simulink model "r2_c8n856m1k_vacc"  (R2013a)
settings: Solver=FixedStepDiscrete  SolverMode=SingleTasking  StartTime=0.0  StopTime=10000  RelTol=1e-3
undefined symbols (not defined in the file): Down, Up
libraries: xbsIndex_r4 x32309, casper_library_misc x83, casper_library_delays x13, casper_library_communications x8, xbsReplacements_r4 x3, xps_library x2
(part 49 of 251)

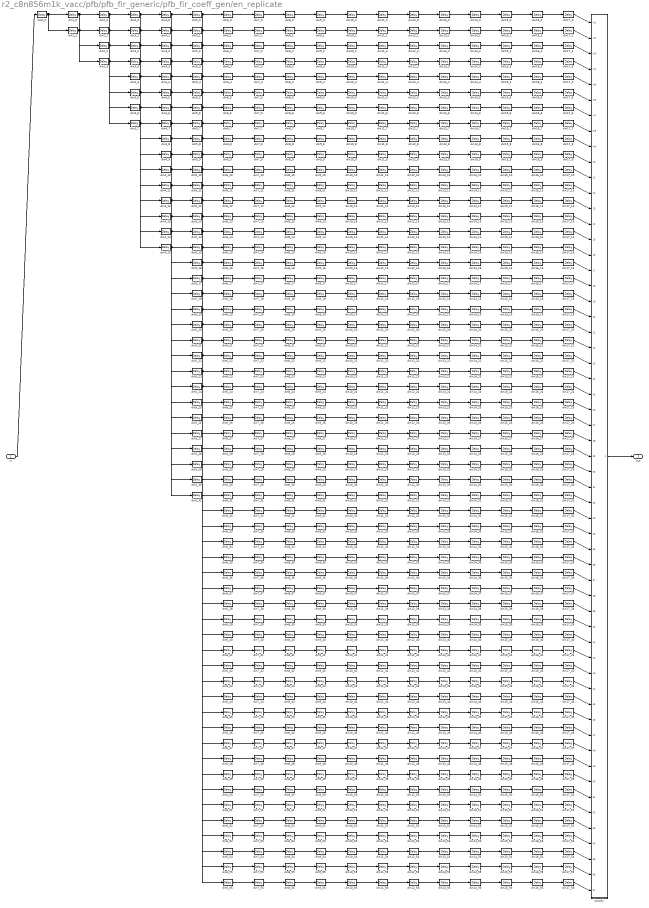
== system r2_c8n856m1k_vacc/pfb/pfb_fir_generic/pfb_fir_coeff_gen/en_replicate ==
  in : Inport
  bussify : Subsystem  AncestorBlock=casper_library_flow_control/bus_create  mask(bus_create){inputNum=57}
      mask prompts: inputNum "Number of inputs:"
      mask init:
      | bus_create_init(gcb,...
      |     'inputNum', inputNum);
  din0_0 : xbsIndex_r4/Delay  rst=off  infoeditControl=Selection of Reset will increase slice count due to use of real FFs and instead of SRLs  en=off  latency=1  dbl_ovrd=off  reg_retiming=off  block_type=delay
  din10_{0..56} (57 alike) : xbsIndex_r4/Delay  rst=off  infoeditControl=Selection of Reset will increase slice count due to use of real FFs and instead of SRLs  en=off  latency=1  dbl_ovrd=off  reg_retiming=on  block_type=delay
  din11_{0..56} (57 alike) : xbsIndex_r4/Delay  rst=off  infoeditControl=Selection of Reset will increase slice count due to use of real FFs and instead of SRLs  en=off  latency=1  dbl_ovrd=off  reg_retiming=on  block_type=delay
  din12_{0..56} (57 alike) : xbsIndex_r4/Delay  rst=off  infoeditControl=Selection of Reset will increase slice count due to use of real FFs and instead of SRLs  en=off  latency=1  dbl_ovrd=off  reg_retiming=on  block_type=delay
  din13_{0..56} (57 alike) : xbsIndex_r4/Delay  rst=off  infoeditControl=Selection of Reset will increase slice count due to use of real FFs and instead of SRLs  en=off  latency=1  dbl_ovrd=off  reg_retiming=on  block_type=delay
  din14_{0..56} (57 alike) : xbsIndex_r4/Delay  rst=off  infoeditControl=Selection of Reset will increase slice count due to use of real FFs and instead of SRLs  en=off  latency=1  dbl_ovrd=off  reg_retiming=on  block_type=delay
  din15_{0..56} (57 alike) : xbsIndex_r4/Delay  rst=off  infoeditControl=Selection of Reset will increase slice count due to use of real FFs and instead of SRLs  en=off  latency=1  dbl_ovrd=off  reg_retiming=on  block_type=delay
  din16_{0..56} (57 alike) : xbsIndex_r4/Delay  rst=off  infoeditControl=Selection of Reset will increase slice count due to use of real FFs and instead of SRLs  en=off  latency=1  dbl_ovrd=off  reg_retiming=on  block_type=delay
  din17_{0..56} (57 alike) : xbsIndex_r4/Delay  rst=off  infoeditControl=Selection of Reset will increase slice count due to use of real FFs and instead of SRLs  en=off  latency=1  dbl_ovrd=off  reg_retiming=off  block_type=delay
  din1_0 : xbsIndex_r4/Delay  rst=off  infoeditControl=Selection of Reset will increase slice count due to use of real FFs and instead of SRLs  en=off  latency=1  dbl_ovrd=off  reg_retiming=off  block_type=delay
  din1_1 : xbsIndex_r4/Delay  rst=off  infoeditControl=Selection of Reset will increase slice count due to use of real FFs and instead of SRLs  en=off  latency=1  dbl_ovrd=off  reg_retiming=off  block_type=delay
  din2_{0..3} (4 alike) : xbsIndex_r4/Delay  rst=off  infoeditControl=Selection of Reset will increase slice count due to use of real FFs and instead of SRLs  en=off  latency=1  dbl_ovrd=off  reg_retiming=off  block_type=delay
  din3_{0..7} (8 alike) : xbsIndex_r4/Delay  rst=off  infoeditControl=Selection of Reset will increase slice count due to use of real FFs and instead of SRLs  en=off  latency=1  dbl_ovrd=off  reg_retiming=off  block_type=delay
  din4_{0..15} (16 alike) : xbsIndex_r4/Delay  rst=off  infoeditControl=Selection of Reset will increase slice count due to use of real FFs and instead of SRLs  en=off  latency=1  dbl_ovrd=off  reg_retiming=off  block_type=delay
  din5_{0..31} (32 alike) : xbsIndex_r4/Delay  rst=off  infoeditControl=Selection of Reset will increase slice count due to use of real FFs and instead of SRLs  en=off  latency=1  dbl_ovrd=off  reg_retiming=off  block_type=delay
  din6_{0..56} (57 alike) : xbsIndex_r4/Delay  rst=off  infoeditControl=Selection of Reset will increase slice count due to use of real FFs and instead of SRLs  en=off  latency=1  dbl_ovrd=off  reg_retiming=on  block_type=delay
  din7_{0..56} (57 alike) : xbsIndex_r4/Delay  rst=off  infoeditControl=Selection of Reset will increase slice count due to use of real FFs and instead of SRLs  en=off  latency=1  dbl_ovrd=off  reg_retiming=on  block_type=delay
  din8_{0..56} (57 alike) : xbsIndex_r4/Delay  rst=off  infoeditControl=Selection of Reset will increase slice count due to use of real FFs and instead of SRLs  en=off  latency=1  dbl_ovrd=off  reg_retiming=on  block_type=delay
  din9_{0..56} (57 alike) : xbsIndex_r4/Delay  rst=off  infoeditControl=Selection of Reset will increase slice count due to use of real FFs and instead of SRLs  en=off  latency=1  dbl_ovrd=off  reg_retiming=on  block_type=delay
  out : Outport
  wires:
  in/1 -> din0_0/1
  din0_0/1 -> din1_0/1, din1_1/1
  din1_0/1 -> din2_0/1, din2_2/1
  din1_1/1 -> din2_1/1, din2_3/1
  din2_0/1 -> din3_0/1, din3_4/1
  din2_1/1 -> din3_1/1, din3_5/1
  din2_2/1 -> din3_2/1, din3_6/1
  din2_3/1 -> din3_3/1, din3_7/1
  din3_0/1 -> din4_0/1, din4_8/1
  din3_1/1 -> din4_1/1, din4_9/1
  din3_2/1 -> din4_2/1, din4_10/1
  din3_3/1 -> din4_3/1, din4_11/1
  din3_4/1 -> din4_4/1, din4_12/1
  din3_5/1 -> din4_5/1, din4_13/1
  din3_6/1 -> din4_6/1, din4_14/1
  din3_7/1 -> din4_7/1, din4_15/1
  din4_0/1 -> din5_0/1, din5_16/1
  din4_1/1 -> din5_1/1, din5_17/1
  din4_2/1 -> din5_2/1, din5_18/1
  din4_3/1 -> din5_3/1, din5_19/1
  din4_4/1 -> din5_4/1, din5_20/1
  din4_5/1 -> din5_5/1, din5_21/1
  din4_6/1 -> din5_6/1, din5_22/1
  din4_7/1 -> din5_7/1, din5_23/1
  din4_8/1 -> din5_8/1, din5_24/1
  din4_9/1 -> din5_9/1, din5_25/1
  din4_10/1 -> din5_10/1, din5_26/1
  din4_11/1 -> din5_11/1, din5_27/1
  din4_12/1 -> din5_12/1, din5_28/1
  din4_13/1 -> din5_13/1, din5_29/1
  din4_14/1 -> din5_14/1, din5_30/1
  din4_15/1 -> din5_15/1, din5_31/1
  din5_0/1 -> din6_0/1, din6_32/1
  din5_1/1 -> din6_1/1, din6_33/1
  din5_2/1 -> din6_2/1, din6_34/1
  din5_3/1 -> din6_3/1, din6_35/1
  din5_4/1 -> din6_4/1, din6_36/1
  din5_5/1 -> din6_5/1, din6_37/1
  din5_6/1 -> din6_6/1, din6_38/1
  din5_7/1 -> din6_7/1, din6_39/1
  din5_8/1 -> din6_8/1, din6_40/1
  din5_9/1 -> din6_9/1, din6_41/1
  din5_10/1 -> din6_10/1, din6_42/1
  din5_11/1 -> din6_11/1, din6_43/1
  din5_12/1 -> din6_12/1, din6_44/1
  din5_13/1 -> din6_13/1, din6_45/1
  din5_14/1 -> din6_14/1, din6_46/1
  din5_15/1 -> din6_15/1, din6_47/1
  din5_16/1 -> din6_16/1, din6_48/1
  din5_17/1 -> din6_17/1, din6_49/1
  din5_18/1 -> din6_18/1, din6_50/1
  din5_19/1 -> din6_19/1, din6_51/1
  din5_20/1 -> din6_20/1, din6_52/1
  din5_21/1 -> din6_21/1, din6_53/1
  din5_22/1 -> din6_22/1, din6_54/1
  din5_23/1 -> din6_23/1, din6_55/1
  din5_24/1 -> din6_24/1, din6_56/1
  din5_25/1 -> din6_25/1
  din5_26/1 -> din6_26/1
  din5_27/1 -> din6_27/1
  din5_28/1 -> din6_28/1
  din5_29/1 -> din6_29/1
  din5_30/1 -> din6_30/1
  din5_31/1 -> din6_31/1
  din6_0/1 -> din7_0/1
  din6_1/1 -> din7_1/1
  din6_2/1 -> din7_2/1
  din6_3/1 -> din7_3/1
  din6_4/1 -> din7_4/1
  din6_5/1 -> din7_5/1
  din6_6/1 -> din7_6/1
  din6_7/1 -> din7_7/1
  din6_8/1 -> din7_8/1
  din6_9/1 -> din7_9/1
  din6_10/1 -> din7_10/1
  din6_11/1 -> din7_11/1
  din6_12/1 -> din7_12/1
  din6_13/1 -> din7_13/1
  din6_14/1 -> din7_14/1
  din6_15/1 -> din7_15/1
  din6_16/1 -> din7_16/1
  din6_17/1 -> din7_17/1
  din6_18/1 -> din7_18/1
  din6_19/1 -> din7_19/1
  din6_20/1 -> din7_20/1
  din6_21/1 -> din7_21/1
  din6_22/1 -> din7_22/1
  din6_23/1 -> din7_23/1
  din6_24/1 -> din7_24/1
  din6_25/1 -> din7_25/1
  din6_26/1 -> din7_26/1
  din6_27/1 -> din7_27/1
  din6_28/1 -> din7_28/1
  din6_29/1 -> din7_29/1
  din6_30/1 -> din7_30/1
  din6_31/1 -> din7_31/1
  din6_32/1 -> din7_32/1
  din6_33/1 -> din7_33/1
  din6_34/1 -> din7_34/1
  din6_35/1 -> din7_35/1
  din6_36/1 -> din7_36/1
  din6_37/1 -> din7_37/1
  din6_38/1 -> din7_38/1
  din6_39/1 -> din7_39/1
  din6_40/1 -> din7_40/1
  din6_41/1 -> din7_41/1
  din6_42/1 -> din7_42/1
  din6_43/1 -> din7_43/1
  din6_44/1 -> din7_44/1
  din6_45/1 -> din7_45/1
  din6_46/1 -> din7_46/1
  din6_47/1 -> din7_47/1
  din6_48/1 -> din7_48/1
  din6_49/1 -> din7_49/1
  din6_50/1 -> din7_50/1
  din6_51/1 -> din7_51/1
  din6_52/1 -> din7_52/1
  din6_53/1 -> din7_53/1
  din6_54/1 -> din7_54/1
  din6_55/1 -> din7_55/1
  din6_56/1 -> din7_56/1
  din7_0/1 -> din8_0/1
  din7_1/1 -> din8_1/1
  din7_2/1 -> din8_2/1
  din7_3/1 -> din8_3/1
  din7_4/1 -> din8_4/1
  din7_5/1 -> din8_5/1
  din7_6/1 -> din8_6/1
  din7_7/1 -> din8_7/1
  din7_8/1 -> din8_8/1
  din7_9/1 -> din8_9/1
  din7_10/1 -> din8_10/1
  din7_11/1 -> din8_11/1
  din7_12/1 -> din8_12/1
  din7_13/1 -> din8_13/1
  din7_14/1 -> din8_14/1
  din7_15/1 -> din8_15/1
  din7_16/1 -> din8_16/1
  din7_17/1 -> din8_17/1
  din7_18/1 -> din8_18/1
  din7_19/1 -> din8_19/1
  din7_20/1 -> din8_20/1
  din7_21/1 -> din8_21/1
  din7_22/1 -> din8_22/1
  din7_23/1 -> din8_23/1
  din7_24/1 -> din8_24/1
  din7_25/1 -> din8_25/1
  din7_26/1 -> din8_26/1
  din7_27/1 -> din8_27/1
  din7_28/1 -> din8_28/1
  din7_29/1 -> din8_29/1
  din7_30/1 -> din8_30/1
  din7_31/1 -> din8_31/1
  din7_32/1 -> din8_32/1
  din7_33/1 -> din8_33/1
  din7_34/1 -> din8_34/1
  din7_35/1 -> din8_35/1
  din7_36/1 -> din8_36/1
  din7_37/1 -> din8_37/1
  din7_38/1 -> din8_38/1
  din7_39/1 -> din8_39/1
  din7_40/1 -> din8_40/1
  din7_41/1 -> din8_41/1
  din7_42/1 -> din8_42/1
  din7_43/1 -> din8_43/1
  din7_44/1 -> din8_44/1
  din7_45/1 -> din8_45/1
  din7_46/1 -> din8_46/1
  din7_47/1 -> din8_47/1
  din7_48/1 -> din8_48/1
  din7_49/1 -> din8_49/1
  din7_50/1 -> din8_50/1
  din7_51/1 -> din8_51/1
  din7_52/1 -> din8_52/1
  din7_53/1 -> din8_53/1
  din7_54/1 -> din8_54/1
  din7_55/1 -> din8_55/1
  din7_56/1 -> din8_56/1
  din8_0/1 -> din9_0/1
  din8_1/1 -> din9_1/1
  din8_2/1 -> din9_2/1
  din8_3/1 -> din9_3/1
  din8_4/1 -> din9_4/1
  din8_5/1 -> din9_5/1
  din8_6/1 -> din9_6/1
  din8_7/1 -> din9_7/1
  din8_8/1 -> din9_8/1
  din8_9/1 -> din9_9/1
  din8_10/1 -> din9_10/1
  din8_11/1 -> din9_11/1
  din8_12/1 -> din9_12/1
  din8_13/1 -> din9_13/1
  din8_14/1 -> din9_14/1
  din8_15/1 -> din9_15/1
  din8_16/1 -> din9_16/1
  din8_17/1 -> din9_17/1
  din8_18/1 -> din9_18/1
  din8_19/1 -> din9_19/1
  din8_20/1 -> din9_20/1
  din8_21/1 -> din9_21/1
  din8_22/1 -> din9_22/1
  din8_23/1 -> din9_23/1
  din8_24/1 -> din9_24/1
  din8_25/1 -> din9_25/1
  din8_26/1 -> din9_26/1
  din8_27/1 -> din9_27/1
  din8_28/1 -> din9_28/1
  din8_29/1 -> din9_29/1
  din8_30/1 -> din9_30/1
  din8_31/1 -> din9_31/1
  din8_32/1 -> din9_32/1
  din8_33/1 -> din9_33/1
  din8_34/1 -> din9_34/1
  din8_35/1 -> din9_35/1
  din8_36/1 -> din9_36/1
  din8_37/1 -> din9_37/1
  din8_38/1 -> din9_38/1
  din8_39/1 -> din9_39/1
  din8_40/1 -> din9_40/1
  din8_41/1 -> din9_41/1
  din8_42/1 -> din9_42/1
  din8_43/1 -> din9_43/1
  din8_44/1 -> din9_44/1
  din8_45/1 -> din9_45/1
  din8_46/1 -> din9_46/1
  din8_47/1 -> din9_47/1
  din8_48/1 -> din9_48/1
  din8_49/1 -> din9_49/1
  din8_50/1 -> din9_50/1
  din8_51/1 -> din9_51/1
  din8_52/1 -> din9_52/1
  din8_53/1 -> din9_53/1
  din8_54/1 -> din9_54/1
  din8_55/1 -> din9_55/1
  din8_56/1 -> din9_56/1
  din9_0/1 -> din10_0/1
  din9_1/1 -> din10_1/1
  din9_2/1 -> din10_2/1
  din9_3/1 -> din10_3/1
  din9_4/1 -> din10_4/1
  din9_5/1 -> din10_5/1
  din9_6/1 -> din10_6/1
  din9_7/1 -> din10_7/1
  din9_8/1 -> din10_8/1
  din9_9/1 -> din10_9/1
  din9_10/1 -> din10_10/1
  din9_11/1 -> din10_11/1
  din9_12/1 -> din10_12/1
  din9_13/1 -> din10_13/1
  din9_14/1 -> din10_14/1
  din9_15/1 -> din10_15/1
  din9_16/1 -> din10_16/1
  din9_17/1 -> din10_17/1
  din9_18/1 -> din10_18/1
  din9_19/1 -> din10_19/1
  din9_20/1 -> din10_20/1
  din9_21/1 -> din10_21/1
  din9_22/1 -> din10_22/1
  din9_23/1 -> din10_23/1
  din9_24/1 -> din10_24/1
  din9_25/1 -> din10_25/1
  din9_26/1 -> din10_26/1
  din9_27/1 -> din10_27/1
  din9_28/1 -> din10_28/1
  din9_29/1 -> din10_29/1
  din9_30/1 -> din10_30/1
  din9_31/1 -> din10_31/1
  din9_32/1 -> din10_32/1
  din9_33/1 -> din10_33/1
  din9_34/1 -> din10_34/1
  din9_35/1 -> din10_35/1
  din9_36/1 -> din10_36/1
  din9_37/1 -> din10_37/1
  din9_38/1 -> din10_38/1
  din9_39/1 -> din10_39/1
  din9_40/1 -> din10_40/1
  din9_41/1 -> din10_41/1
  din9_42/1 -> din10_42/1
  din9_43/1 -> din10_43/1
  din9_44/1 -> din10_44/1
  din9_45/1 -> din10_45/1
  din9_46/1 -> din10_46/1
  din9_47/1 -> din10_47/1
  din9_48/1 -> din10_48/1
  din9_49/1 -> din10_49/1
  din9_50/1 -> din10_50/1
  din9_51/1 -> din10_51/1
  din9_52/1 -> din10_52/1
  din9_53/1 -> din10_53/1
  din9_54/1 -> din10_54/1
  din9_55/1 -> din10_55/1
  din9_56/1 -> din10_56/1
  din10_0/1 -> din11_0/1
  din10_1/1 -> din11_1/1
  din10_2/1 -> din11_2/1
  din10_3/1 -> din11_3/1
  din10_4/1 -> din11_4/1
  din10_5/1 -> din11_5/1
  din10_6/1 -> din11_6/1
  din10_7/1 -> din11_7/1
  din10_8/1 -> din11_8/1
  din10_9/1 -> din11_9/1
  din10_10/1 -> din11_10/1
  din10_11/1 -> din11_11/1
  din10_12/1 -> din11_12/1
  din10_13/1 -> din11_13/1
  din10_14/1 -> din11_14/1
  din10_15/1 -> din11_15/1
  din10_16/1 -> din11_16/1
  din10_17/1 -> din11_17/1
  din10_18/1 -> din11_18/1
  din10_19/1 -> din11_19/1
  din10_20/1 -> din11_20/1
  din10_21/1 -> din11_21/1
  din10_22/1 -> din11_22/1
  din10_23/1 -> din11_23/1
  din10_24/1 -> din11_24/1
  din10_25/1 -> din11_25/1
  din10_26/1 -> din11_26/1
  din10_27/1 -> din11_27/1
  din10_28/1 -> din11_28/1
  din10_29/1 -> din11_29/1
  din10_30/1 -> din11_30/1
  din10_31/1 -> din11_31/1
  din10_32/1 -> din11_32/1
  din10_33/1 -> din11_33/1
  din10_34/1 -> din11_34/1
  din10_35/1 -> din11_35/1
  din10_36/1 -> din11_36/1
  din10_37/1 -> din11_37/1
  din10_38/1 -> din11_38/1
  din10_39/1 -> din11_39/1
  din10_40/1 -> din11_40/1
  din10_41/1 -> din11_41/1
  din10_42/1 -> din11_42/1
  din10_43/1 -> din11_43/1
  din10_44/1 -> din11_44/1
  din10_45/1 -> din11_45/1
  din10_46/1 -> din11_46/1
  din10_47/1 -> din11_47/1
  din10_48/1 -> din11_48/1
  din10_49/1 -> din11_49/1
  din10_50/1 -> din11_50/1
  din10_51/1 -> din11_51/1
  din10_52/1 -> din11_52/1
  din10_53/1 -> din11_53/1
  din10_54/1 -> din11_54/1
  din10_55/1 -> din11_55/1
  din10_56/1 -> din11_56/1
  din11_0/1 -> din12_0/1
  din11_1/1 -> din12_1/1
  din11_2/1 -> din12_2/1
  din11_3/1 -> din12_3/1
  din11_4/1 -> din12_4/1
  din11_5/1 -> din12_5/1
  din11_6/1 -> din12_6/1
  din11_7/1 -> din12_7/1
  din11_8/1 -> din12_8/1
  din11_9/1 -> din12_9/1
  din11_10/1 -> din12_10/1
  din11_11/1 -> din12_11/1
  din11_12/1 -> din12_12/1
  din11_13/1 -> din12_13/1
  din11_14/1 -> din12_14/1
  din11_15/1 -> din12_15/1
  din11_16/1 -> din12_16/1
  din11_17/1 -> din12_17/1
  din11_18/1 -> din12_18/1
  din11_19/1 -> din12_19/1
  din11_20/1 -> din12_20/1
  din11_21/1 -> din12_21/1
  din11_22/1 -> din12_22/1
  din11_23/1 -> din12_23/1
  din11_24/1 -> din12_24/1
  din11_25/1 -> din12_25/1
  din11_26/1 -> din12_26/1
  din11_27/1 -> din12_27/1
  din11_28/1 -> din12_28/1
  din11_29/1 -> din12_29/1
  din11_30/1 -> din12_30/1
  din11_31/1 -> din12_31/1
  din11_32/1 -> din12_32/1
  din11_33/1 -> din12_33/1
  din11_34/1 -> din12_34/1
  din11_35/1 -> din12_35/1
  din11_36/1 -> din12_36/1
  din11_37/1 -> din12_37/1
  din11_38/1 -> din12_38/1
  din11_39/1 -> din12_39/1
  din11_40/1 -> din12_40/1
  din11_41/1 -> din12_41/1
  din11_42/1 -> din12_42/1
  din11_43/1 -> din12_43/1
  din11_44/1 -> din12_44/1
  din11_45/1 -> din12_45/1
  din11_46/1 -> din12_46/1
  din11_47/1 -> din12_47/1
  din11_48/1 -> din12_48/1
  din11_49/1 -> din12_49/1
  din11_50/1 -> din12_50/1
  din11_51/1 -> din12_51/1
  din11_52/1 -> din12_52/1
  din11_53/1 -> din12_53/1
  din11_54/1 -> din12_54/1
  din11_55/1 -> din12_55/1
  din11_56/1 -> din12_56/1
  din12_0/1 -> din13_0/1
  din12_1/1 -> din13_1/1
  din12_2/1 -> din13_2/1
  din12_3/1 -> din13_3/1
  din12_4/1 -> din13_4/1
  din12_5/1 -> din13_5/1
  din12_6/1 -> din13_6/1
  din12_7/1 -> din13_7/1
  din12_8/1 -> din13_8/1
  din12_9/1 -> din13_9/1
  din12_10/1 -> din13_10/1
  din12_11/1 -> din13_11/1
  din12_12/1 -> din13_12/1
  din12_13/1 -> din13_13/1
  din12_14/1 -> din13_14/1
  din12_15/1 -> din13_15/1
  din12_16/1 -> din13_16/1
  din12_17/1 -> din13_17/1
  din12_18/1 -> din13_18/1
  din12_19/1 -> din13_19/1
  din12_20/1 -> din13_20/1
  din12_21/1 -> din13_21/1
  din12_22/1 -> din13_22/1
  din12_23/1 -> din13_23/1
  din12_24/1 -> din13_24/1
  din12_25/1 -> din13_25/1
  din12_26/1 -> din13_26/1
  din12_27/1 -> din13_27/1
  din12_28/1 -> din13_28/1
  din12_29/1 -> din13_29/1
  din12_30/1 -> din13_30/1
  din12_31/1 -> din13_31/1
  din12_32/1 -> din13_32/1
  din12_33/1 -> din13_33/1
  din12_34/1 -> din13_34/1
  din12_35/1 -> din13_35/1
  din12_36/1 -> din13_36/1
  din12_37/1 -> din13_37/1
  din12_38/1 -> din13_38/1
  din12_39/1 -> din13_39/1
  din12_40/1 -> din13_40/1
  din12_41/1 -> din13_41/1
  din12_42/1 -> din13_42/1
  din12_43/1 -> din13_43/1
  din12_44/1 -> din13_44/1
  din12_45/1 -> din13_45/1
  din12_46/1 -> din13_46/1
  din12_47/1 -> din13_47/1
  din12_48/1 -> din13_48/1
  din12_49/1 -> din13_49/1
  din12_50/1 -> din13_50/1
  din12_51/1 -> din13_51/1
  din12_52/1 -> din13_52/1
  din12_53/1 -> din13_53/1
  din12_54/1 -> din13_54/1
  din12_55/1 -> din13_55/1
  din12_56/1 -> din13_56/1
  din13_0/1 -> din14_0/1
  din13_1/1 -> din14_1/1
  din13_2/1 -> din14_2/1
  din13_3/1 -> din14_3/1
  din13_4/1 -> din14_4/1
  din13_5/1 -> din14_5/1
  din13_6/1 -> din14_6/1
  din13_7/1 -> din14_7/1
  din13_8/1 -> din14_8/1
  din13_9/1 -> din14_9/1
  din13_10/1 -> din14_10/1
  din13_11/1 -> din14_11/1
  din13_12/1 -> din14_12/1
  din13_13/1 -> din14_13/1
  din13_14/1 -> din14_14/1
  din13_15/1 -> din14_15/1
  din13_16/1 -> din14_16/1
  din13_17/1 -> din14_17/1
  din13_18/1 -> din14_18/1
  din13_19/1 -> din14_19/1
  din13_20/1 -> din14_20/1
  din13_21/1 -> din14_21/1
  din13_22/1 -> din14_22/1
  din13_23/1 -> din14_23/1
  din13_24/1 -> din14_24/1
  din13_25/1 -> din14_25/1
  din13_26/1 -> din14_26/1
  din13_27/1 -> din14_27/1
  din13_28/1 -> din14_28/1
  din13_29/1 -> din14_29/1
  din13_30/1 -> din14_30/1
  din13_31/1 -> din14_31/1
  din13_32/1 -> din14_32/1
  din13_33/1 -> din14_33/1
  din13_34/1 -> din14_34/1
  din13_35/1 -> din14_35/1
  din13_36/1 -> din14_36/1
  din13_37/1 -> din14_37/1
  din13_38/1 -> din14_38/1
  din13_39/1 -> din14_39/1
  din13_40/1 -> din14_40/1
  din13_41/1 -> din14_41/1
  din13_42/1 -> din14_42/1
  din13_43/1 -> din14_43/1
  din13_44/1 -> din14_44/1
  din13_45/1 -> din14_45/1
  din13_46/1 -> din14_46/1
  din13_47/1 -> din14_47/1
  din13_48/1 -> din14_48/1
  din13_49/1 -> din14_49/1
  din13_50/1 -> din14_50/1
  din13_51/1 -> din14_51/1
  din13_52/1 -> din14_52/1
  din13_53/1 -> din14_53/1
  din13_54/1 -> din14_54/1
  din13_55/1 -> din14_55/1
  din13_56/1 -> din14_56/1
  din14_0/1 -> din15_0/1
  din14_1/1 -> din15_1/1
  din14_2/1 -> din15_2/1
  din14_3/1 -> din15_3/1
  din14_4/1 -> din15_4/1
  din14_5/1 -> din15_5/1
  din14_6/1 -> din15_6/1
  din14_7/1 -> din15_7/1
  din14_8/1 -> din15_8/1
  din14_9/1 -> din15_9/1
  din14_10/1 -> din15_10/1
  din14_11/1 -> din15_11/1
  din14_12/1 -> din15_12/1
  din14_13/1 -> din15_13/1
  din14_14/1 -> din15_14/1
  din14_15/1 -> din15_15/1
  din14_16/1 -> din15_16/1
  din14_17/1 -> din15_17/1
  din14_18/1 -> din15_18/1
  din14_19/1 -> din15_19/1
  din14_20/1 -> din15_20/1
  din14_21/1 -> din15_21/1
  din14_22/1 -> din15_22/1
  din14_23/1 -> din15_23/1
  din14_24/1 -> din15_24/1
  din14_25/1 -> din15_25/1
  din14_26/1 -> din15_26/1
  din14_27/1 -> din15_27/1
  din14_28/1 -> din15_28/1
  din14_29/1 -> din15_29/1
  din14_30/1 -> din15_30/1
  din14_31/1 -> din15_31/1
  din14_32/1 -> din15_32/1
  din14_33/1 -> din15_33/1
  din14_34/1 -> din15_34/1
  din14_35/1 -> din15_35/1
  din14_36/1 -> din15_36/1
  din14_37/1 -> din15_37/1
  din14_38/1 -> din15_38/1
  din14_39/1 -> din15_39/1
  din14_40/1 -> din15_40/1
  din14_41/1 -> din15_41/1
  din14_42/1 -> din15_42/1
  din14_43/1 -> din15_43/1
  din14_44/1 -> din15_44/1
  din14_45/1 -> din15_45/1
  din14_46/1 -> din15_46/1
  din14_47/1 -> din15_47/1
  din14_48/1 -> din15_48/1
  din14_49/1 -> din15_49/1
  din14_50/1 -> din15_50/1
  din14_51/1 -> din15_51/1
  din14_52/1 -> din15_52/1
  din14_53/1 -> din15_53/1
  din14_54/1 -> din15_54/1
  din14_55/1 -> din15_55/1
  din14_56/1 -> din15_56/1
  din15_0/1 -> din16_0/1
  din15_1/1 -> din16_1/1
  din15_2/1 -> din16_2/1
  din15_3/1 -> din16_3/1
  din15_4/1 -> din16_4/1
  din15_5/1 -> din16_5/1
  din15_6/1 -> din16_6/1
  din15_7/1 -> din16_7/1
  din15_8/1 -> din16_8/1
  din15_9/1 -> din16_9/1
  din15_10/1 -> din16_10/1
  din15_11/1 -> din16_11/1
  din15_12/1 -> din16_12/1
  din15_13/1 -> din16_13/1
  din15_14/1 -> din16_14/1
  din15_15/1 -> din16_15/1
  din15_16/1 -> din16_16/1
  din15_17/1 -> din16_17/1
  din15_18/1 -> din16_18/1
  din15_19/1 -> din16_19/1
  din15_20/1 -> din16_20/1
  din15_21/1 -> din16_21/1
  din15_22/1 -> din16_22/1
  din15_23/1 -> din16_23/1
  din15_24/1 -> din16_24/1
  din15_25/1 -> din16_25/1
  din15_26/1 -> din16_26/1
  din15_27/1 -> din16_27/1
  din15_28/1 -> din16_28/1
  din15_29/1 -> din16_29/1
  din15_30/1 -> din16_30/1
  din15_31/1 -> din16_31/1
  din15_32/1 -> din16_32/1
  din15_33/1 -> din16_33/1
  din15_34/1 -> din16_34/1
  din15_35/1 -> din16_35/1
  din15_36/1 -> din16_36/1
  din15_37/1 -> din16_37/1
  din15_38/1 -> din16_38/1
  din15_39/1 -> din16_39/1
  din15_40/1 -> din16_40/1
  din15_41/1 -> din16_41/1
  din15_42/1 -> din16_42/1
  din15_43/1 -> din16_43/1
  din15_44/1 -> din16_44/1
  din15_45/1 -> din16_45/1
  din15_46/1 -> din16_46/1
  din15_47/1 -> din16_47/1
  din15_48/1 -> din16_48/1
  din15_49/1 -> din16_49/1
  din15_50/1 -> din16_50/1
  din15_51/1 -> din16_51/1
  din15_52/1 -> din16_52/1
  din15_53/1 -> din16_53/1
  din15_54/1 -> din16_54/1
  din15_55/1 -> din16_55/1
  din15_56/1 -> din16_56/1
  din16_0/1 -> din17_0/1
  din16_1/1 -> din17_1/1
  din16_2/1 -> din17_2/1
  din16_3/1 -> din17_3/1
  din16_4/1 -> din17_4/1
  din16_5/1 -> din17_5/1
  din16_6/1 -> din17_6/1
  din16_7/1 -> din17_7/1
  din16_8/1 -> din17_8/1
  din16_9/1 -> din17_9/1
  din16_10/1 -> din17_10/1
  din16_11/1 -> din17_11/1
  din16_12/1 -> din17_12/1
  din16_13/1 -> din17_13/1
  din16_14/1 -> din17_14/1
  din16_15/1 -> din17_15/1
  din16_16/1 -> din17_16/1
  din16_17/1 -> din17_17/1
  din16_18/1 -> din17_18/1
  din16_19/1 -> din17_19/1
  din16_20/1 -> din17_20/1
  din16_21/1 -> din17_21/1
  din16_22/1 -> din17_22/1
  din16_23/1 -> din17_23/1
  din16_24/1 -> din17_24/1
  din16_25/1 -> din17_25/1
  din16_26/1 -> din17_26/1
  din16_27/1 -> din17_27/1
  din16_28/1 -> din17_28/1
  din16_29/1 -> din17_29/1
  din16_30/1 -> din17_30/1
  din16_31/1 -> din17_31/1
  din16_32/1 -> din17_32/1
  din16_33/1 -> din17_33/1
  din16_34/1 -> din17_34/1
  din16_35/1 -> din17_35/1
  din16_36/1 -> din17_36/1
  din16_37/1 -> din17_37/1
  din16_38/1 -> din17_38/1
  din16_39/1 -> din17_39/1
  din16_40/1 -> din17_40/1
  din16_41/1 -> din17_41/1
  din16_42/1 -> din17_42/1
  din16_43/1 -> din17_43/1
  din16_44/1 -> din17_44/1
  din16_45/1 -> din17_45/1
  din16_46/1 -> din17_46/1
  din16_47/1 -> din17_47/1
  din16_48/1 -> din17_48/1
  din16_49/1 -> din17_49/1
  din16_50/1 -> din17_50/1
  din16_51/1 -> din17_51/1
  din16_52/1 -> din17_52/1
  din16_53/1 -> din17_53/1
  din16_54/1 -> din17_54/1
  din16_55/1 -> din17_55/1
  din16_56/1 -> din17_56/1
  din17_0/1 -> bussify/1(in1)
  din17_1/1 -> bussify/2(in2)
  din17_2/1 -> bussify/3(in3)
  din17_3/1 -> bussify/4(in4)
  din17_4/1 -> bussify/5(in5)
  din17_5/1 -> bussify/6(in6)
  din17_6/1 -> bussify/7(in7)
  din17_7/1 -> bussify/8(in8)
  din17_8/1 -> bussify/9(in9)
  din17_9/1 -> bussify/10(in10)
  din17_10/1 -> bussify/11(in11)
  din17_11/1 -> bussify/12(in12)
  din17_12/1 -> bussify/13(in13)
  din17_13/1 -> bussify/14(in14)
  din17_14/1 -> bussify/15(in15)
  din17_15/1 -> bussify/16(in16)
  din17_16/1 -> bussify/17(in17)
  din17_17/1 -> bussify/18(in18)
  din17_18/1 -> bussify/19(in19)
  din17_19/1 -> bussify/20(in20)
  din17_20/1 -> bussify/21(in21)
  din17_21/1 -> bussify/22(in22)
  din17_22/1 -> bussify/23(in23)
  din17_23/1 -> bussify/24(in24)
  din17_24/1 -> bussify/25(in25)
  din17_25/1 -> bussify/26(in26)
  din17_26/1 -> bussify/27(in27)
  din17_27/1 -> bussify/28(in28)
  din17_28/1 -> bussify/29(in29)
  din17_29/1 -> bussify/30(in30)
  din17_30/1 -> bussify/31(in31)
  din17_31/1 -> bussify/32(in32)
  din17_32/1 -> bussify/33(in33)
  din17_33/1 -> bussify/34(in34)
  din17_34/1 -> bussify/35(in35)
  din17_35/1 -> bussify/36(in36)
  din17_36/1 -> bussify/37(in37)
  din17_37/1 -> bussify/38(in38)
  din17_38/1 -> bussify/39(in39)
  din17_39/1 -> bussify/40(in40)
  din17_40/1 -> bussify/41(in41)
  din17_41/1 -> bussify/42(in42)
  din17_42/1 -> bussify/43(in43)
  din17_43/1 -> bussify/44(in44)
  din17_44/1 -> bussify/45(in45)
  din17_45/1 -> bussify/46(in46)
  din17_46/1 -> bussify/47(in47)
  din17_47/1 -> bussify/48(in48)
  din17_48/1 -> bussify/49(in49)
  din17_49/1 -> bussify/50(in50)
  din17_50/1 -> bussify/51(in51)
  din17_51/1 -> bussify/52(in52)
  din17_52/1 -> bussify/53(in53)
  din17_53/1 -> bussify/54(in54)
  din17_54/1 -> bussify/55(in55)
  din17_55/1 -> bussify/56(in56)
  din17_56/1 -> bussify/57(in57)
  bussify/1(bus_out) -> out/1

== system r2_c8n856m1k_vacc/pfb/pfb_fir_generic/pfb_fir_coeff_gen/munge ==
  din : Inport
  join : Subsystem  AncestorBlock=casper_library_flow_control/bus_create  mask(bus_create){inputNum=32}
      mask prompts: inputNum "Number of inputs:"
      mask init:
      | bus_create_init(gcb,...
      |     'inputNum', inputNum);
  reinterpret : xbsIndex_r4/Reinterpret  force_arith_type=on  arith_type=Unsigned  force_bin_pt=on  bin_pt=0  block_type=reinterpret
  reinterpret_out : xbsIndex_r4/Reinterpret  force_arith_type=on  arith_type=Unsigned  force_bin_pt=on  bin_pt=0  block_type=reinterpret
  split : Subsystem  AncestorBlock=casper_library_flow_control/bus_expand  mask(bus_expand){mode=divisions of arbitrary size, outputNum=1, outputWidth=[18 18 18 18 18 18 18 18 18 18 18 18 18 18 18 18 18 18 18 18 18 18 18 18 18 18 18 18 18 18 18 18], outputBinaryPt=[0 0 0 0 0 0 0 0 0 0 0 0 0 0 0 0 0 0 0 0 0 0 0 0 0 0 0 0 0 0 0 0], outputArithmeticType=[0 0 0 0 0 0 0 0 0 0 0 0 0 0 0 0 0 0 0 0 0 0 0 0 0 0 0 0 0 0 0 0], show_format=off, outputToWorkspace=off, variablePrefix=out, outputToModelAsWell=on}
      mask prompts: outputNum "Number of outputs:"; outputWidth "Output width [msb ... lsb]:"; outputBinaryPt "Output binary point position [msb ... lsb]:"; outputArithmeticType "Output arithmetic type (ufix=0, fix=1, bool=2) [msb ... lsb]:"; show_format "Print format string?"; outputToWorkspace "Output to workspace?"; variablePrefix "Variable name prefix:"; outputToModelAsWell "Output to model as well?"
      mask help:
      | Expands a 'bus' made using a bus_create or Xilinx
      | concat block.
      | The input will be divided into the specified number
      | of outputs with the specified arithmetic types.
      | Divisions are made from most significant bit.
      mask init: same as r2_c8n856m1k_vacc/bus_expand15
      settings: AttributesFormatString=32 outputs
      mask TypeOptions mode: divisions of equal size | divisions of arbitrary size
      mask Callback mode: bus_expand_callback();
      mask Callback outputWidth: bus_expand_callback();
      mask Callback outputToWorkspace: bus_expand_callback();
  dout : Outport
  wires:
  reinterpret_out/1 -> dout/1
  din/1 -> reinterpret/1
  reinterpret/1 -> split/1(bus_in)
  join/1(bus_out) -> reinterpret_out/1
  split/25(out8) -> join/1(in1)
  split/32(lsb_out1) -> join/2(in2)
  split/31(out2) -> join/3(in3)
  split/30(out3) -> join/4(in4)
  split/29(out4) -> join/5(in5)
  split/28(out5) -> join/6(in6)
  split/27(out6) -> join/7(in7)
  split/26(out7) -> join/8(in8)
  split/17(out16) -> join/9(in9)
  split/24(out9) -> join/10(in10)
  split/23(out10) -> join/11(in11)
  split/22(out11) -> join/12(in12)
  split/21(out12) -> join/13(in13)
  split/20(out13) -> join/14(in14)
  split/19(out14) -> join/15(in15)
  split/18(out15) -> join/16(in16)
  split/9(out24) -> join/17(in17)
  split/16(out17) -> join/18(in18)
  split/15(out18) -> join/19(in19)
  split/14(out19) -> join/20(in20)
  split/13(out20) -> join/21(in21)
  split/12(out21) -> join/22(in22)
  split/11(out22) -> join/23(in23)
  split/10(out23) -> join/24(in24)
  split/1(msb_out32) -> join/25(in25)
  split/8(out25) -> join/26(in26)
  split/7(out26) -> join/27(in27)
  split/6(out27) -> join/28(in28)
  split/5(out28) -> join/29(in29)
  split/4(out29) -> join/30(in30)
  split/3(out30) -> join/31(in31)
  split/2(out31) -> join/32(in32)

== system r2_c8n856m1k_vacc/pfb/pfb_fir_generic/pfb_fir_coeff_gen/rom ==
  addra : Inport
  dina : Inport  Port=2
  wea : Inport  Port=3
  addrb : Inport  Port=4
  dinb : Inport  Port=5
  web : Inport  Port=6
  A_bussify : Subsystem  AncestorBlock=casper_library_flow_control/bus_create  mask(bus_create){inputNum=16}
      mask prompts: inputNum "Number of inputs:"
      mask init:
      | bus_create_init(gcb,...
      |     'inputNum', inputNum);
  B_bussify : Subsystem  AncestorBlock=casper_library_flow_control/bus_create  mask(bus_create){inputNum=16}
      mask prompts: inputNum "Number of inputs:"
      mask init:
      | bus_create_init(gcb,...
      |     'inputNum', inputNum);
  bram{0..15} (16 alike) : xbsIndex_r4/Dual Port RAM  depth=256  initVector=getfield(get_param(gcb, 'UserData'), 'initVector')  distributed_mem=Block RAM  init_a=0  init_b=0  rst_a=off  rst_b=off  en_a=off  en_b=off  latency=9  write_mode_A=Read Before Write  write_mode_B=Read Before Write  dbl_ovrd=off  optimize=Speed  block_type=dpram
  ddina : xbsIndex_r4/Delay  rst=off  infoeditControl=Selection of Reset will increase slice count due to use of real FFs and instead of SRLs  en=off  latency=0  dbl_ovrd=off  reg_retiming=on  block_type=delay
  ddinb : xbsIndex_r4/Delay  rst=off  infoeditControl=Selection of Reset will increase slice count due to use of real FFs and instead of SRLs  en=off  latency=0  dbl_ovrd=off  reg_retiming=on  block_type=delay
  debus_addra : Subsystem  AncestorBlock=casper_library_flow_control/bus_expand  mask(bus_expand){mode=divisions of equal size, outputNum=16, outputWidth=[8 8 8 8 8 8 8 8 8 8 8 8 8 8 8 8], outputBinaryPt=[0 0 0 0 0 0 0 0 0 0 0 0 0 0 0 0], outputArithmeticType=[0 0 0 0 0 0 0 0 0 0 0 0 0 0 0 0], show_format=on, outputToWorkspace=off, variablePrefix=, outputToModelAsWell=on}
      mask prompts: outputNum "Number of outputs:"; outputWidth "Output width:"; outputBinaryPt "Output binary point position:"; outputArithmeticType "Output arithmetic type (ufix=0, fix=1, bool=2):"; show_format "Print format string?"; outputToWorkspace "Output to workspace?"; variablePrefix "Variable name prefix:"; outputToModelAsWell "Output to model as well?"
      mask help:
      | Expands a 'bus' made using a bus_create or Xilinx
      | concat block.
      | The input will be divided into the specified number
      | of outputs with the specified arithmetic types.
      | Divisions are made from most significant bit.
      mask init: same as r2_c8n856m1k_vacc/bus_expand15
      settings: AttributesFormatString=16 outputs: uf8.0,uf8.0,uf8.0,uf8.0,uf8.0,uf8.0,uf8.0,uf8.0,uf8.0,uf8.0,uf8.0,uf8.0,uf8.0,uf8.0,uf8.0,uf8.0,
      mask TypeOptions mode: divisions of equal size | divisions of arbitrary size
      mask Callback mode: bus_expand_callback();
      mask Callback outputWidth: bus_expand_callback();
      mask Callback outputToWorkspace: bus_expand_callback();
  debus_addrb : Subsystem  AncestorBlock=casper_library_flow_control/bus_expand  mask(bus_expand){mode=divisions of equal size, outputNum=16, outputWidth=[8 8 8 8 8 8 8 8 8 8 8 8 8 8 8 8], outputBinaryPt=[0 0 0 0 0 0 0 0 0 0 0 0 0 0 0 0], outputArithmeticType=[0 0 0 0 0 0 0 0 0 0 0 0 0 0 0 0], show_format=on, outputToWorkspace=off, variablePrefix=, outputToModelAsWell=on}
      mask prompts: outputNum "Number of outputs:"; outputWidth "Output width:"; outputBinaryPt "Output binary point position:"; outputArithmeticType "Output arithmetic type (ufix=0, fix=1, bool=2):"; show_format "Print format string?"; outputToWorkspace "Output to workspace?"; variablePrefix "Variable name prefix:"; outputToModelAsWell "Output to model as well?"
      mask help:
      | Expands a 'bus' made using a bus_create or Xilinx
      | concat block.
      | The input will be divided into the specified number
      | of outputs with the specified arithmetic types.
      | Divisions are made from most significant bit.
      mask init: same as r2_c8n856m1k_vacc/bus_expand15
      settings: AttributesFormatString=16 outputs: uf8.0,uf8.0,uf8.0,uf8.0,uf8.0,uf8.0,uf8.0,uf8.0,uf8.0,uf8.0,uf8.0,uf8.0,uf8.0,uf8.0,uf8.0,uf8.0,
      mask TypeOptions mode: divisions of equal size | divisions of arbitrary size
      mask Callback mode: bus_expand_callback();
      mask Callback outputWidth: bus_expand_callback();
      mask Callback outputToWorkspace: bus_expand_callback();
  debus_dina : Subsystem  AncestorBlock=casper_library_flow_control/bus_expand  mask(bus_expand){mode=divisions of arbitrary size, outputNum=1, outputWidth=[36 36 36 36 36 36 36 36 36 36 36 36 36 36 36 36], outputBinaryPt=[0 0 0 0 0 0 0 0 0 0 0 0 0 0 0 0], outputArithmeticType=[0 0 0 0 0 0 0 0 0 0 0 0 0 0 0 0], show_format=on, outputToWorkspace=off, variablePrefix=, outputToModelAsWell=on}
      mask prompts: outputNum "Number of outputs:"; outputWidth "Output width [msb ... lsb]:"; outputBinaryPt "Output binary point position [msb ... lsb]:"; outputArithmeticType "Output arithmetic type (ufix=0, fix=1, bool=2) [msb ... lsb]:"; show_format "Print format string?"; outputToWorkspace "Output to workspace?"; variablePrefix "Variable name prefix:"; outputToModelAsWell "Output to model as well?"
      mask help:
      | Expands a 'bus' made using a bus_create or Xilinx
      | concat block.
      | The input will be divided into the specified number
      | of outputs with the specified arithmetic types.
      | Divisions are made from most significant bit.
      mask init: same as r2_c8n856m1k_vacc/bus_expand15
      settings: AttributesFormatString=16 outputs: uf36.0,uf36.0,uf36.0,uf36.0,uf36.0,uf36.0,uf36.0,uf36.0,uf36.0,uf36.0,uf36.0,uf36.0,uf36.0,uf36.0,uf36.0,uf36.0,
      mask TypeOptions mode: divisions of equal size | divisions of arbitrary size
      mask Callback mode: bus_expand_callback();
      mask Callback outputWidth: bus_expand_callback();
      mask Callback outputToWorkspace: bus_expand_callback();
  debus_dinb : Subsystem  AncestorBlock=casper_library_flow_control/bus_expand  mask(bus_expand){mode=divisions of arbitrary size, outputNum=1, outputWidth=[36 36 36 36 36 36 36 36 36 36 36 36 36 36 36 36], outputBinaryPt=[0 0 0 0 0 0 0 0 0 0 0 0 0 0 0 0], outputArithmeticType=[0 0 0 0 0 0 0 0 0 0 0 0 0 0 0 0], show_format=on, outputToWorkspace=off, variablePrefix=, outputToModelAsWell=on}
      mask prompts: outputNum "Number of outputs:"; outputWidth "Output width [msb ... lsb]:"; outputBinaryPt "Output binary point position [msb ... lsb]:"; outputArithmeticType "Output arithmetic type (ufix=0, fix=1, bool=2) [msb ... lsb]:"; show_format "Print format string?"; outputToWorkspace "Output to workspace?"; variablePrefix "Variable name prefix:"; outputToModelAsWell "Output to model as well?"
      mask help:
      | Expands a 'bus' made using a bus_create or Xilinx
      | concat block.
      | The input will be divided into the specified number
      | of outputs with the specified arithmetic types.
      | Divisions are made from most significant bit.
      mask init: same as r2_c8n856m1k_vacc/bus_expand15
      settings: AttributesFormatString=16 outputs: uf36.0,uf36.0,uf36.0,uf36.0,uf36.0,uf36.0,uf36.0,uf36.0,uf36.0,uf36.0,uf36.0,uf36.0,uf36.0,uf36.0,uf36.0,uf36.0,
      mask TypeOptions mode: divisions of equal size | divisions of arbitrary size
      mask Callback mode: bus_expand_callback();
      mask Callback outputWidth: bus_expand_callback();
      mask Callback outputToWorkspace: bus_expand_callback();
  debus_wea : Subsystem  AncestorBlock=casper_library_flow_control/bus_expand  mask(bus_expand){mode=divisions of equal size, outputNum=16, outputWidth=[1 1 1 1 1 1 1 1 1 1 1 1 1 1 1 1], outputBinaryPt=[0 0 0 0 0 0 0 0 0 0 0 0 0 0 0 0], outputArithmeticType=[2 2 2 2 2 2 2 2 2 2 2 2 2 2 2 2], show_format=on, outputToWorkspace=off, variablePrefix=, outputToModelAsWell=on}
      mask prompts: outputNum "Number of outputs:"; outputWidth "Output width:"; outputBinaryPt "Output binary point position:"; outputArithmeticType "Output arithmetic type (ufix=0, fix=1, bool=2):"; show_format "Print format string?"; outputToWorkspace "Output to workspace?"; variablePrefix "Variable name prefix:"; outputToModelAsWell "Output to model as well?"
      mask help:
      | Expands a 'bus' made using a bus_create or Xilinx
      | concat block.
      | The input will be divided into the specified number
      | of outputs with the specified arithmetic types.
      | Divisions are made from most significant bit.
      mask init: same as r2_c8n856m1k_vacc/bus_expand15
      settings: AttributesFormatString=16 outputs: b,b,b,b,b,b,b,b,b,b,b,b,b,b,b,b,
      mask TypeOptions mode: divisions of equal size | divisions of arbitrary size
      mask Callback mode: bus_expand_callback();
      mask Callback outputWidth: bus_expand_callback();
      mask Callback outputToWorkspace: bus_expand_callback();
  debus_web : Subsystem  AncestorBlock=casper_library_flow_control/bus_expand  mask(bus_expand){mode=divisions of equal size, outputNum=16, outputWidth=[1 1 1 1 1 1 1 1 1 1 1 1 1 1 1 1], outputBinaryPt=[0 0 0 0 0 0 0 0 0 0 0 0 0 0 0 0], outputArithmeticType=[2 2 2 2 2 2 2 2 2 2 2 2 2 2 2 2], show_format=on, outputToWorkspace=off, variablePrefix=, outputToModelAsWell=on}
      mask prompts: outputNum "Number of outputs:"; outputWidth "Output width:"; outputBinaryPt "Output binary point position:"; outputArithmeticType "Output arithmetic type (ufix=0, fix=1, bool=2):"; show_format "Print format string?"; outputToWorkspace "Output to workspace?"; variablePrefix "Variable name prefix:"; outputToModelAsWell "Output to model as well?"
      mask help:
      | Expands a 'bus' made using a bus_create or Xilinx
      | concat block.
      | The input will be divided into the specified number
      | of outputs with the specified arithmetic types.
      | Divisions are made from most significant bit.
      mask init: same as r2_c8n856m1k_vacc/bus_expand15
      settings: AttributesFormatString=16 outputs: b,b,b,b,b,b,b,b,b,b,b,b,b,b,b,b,
      mask TypeOptions mode: divisions of equal size | divisions of arbitrary size
      mask Callback mode: bus_expand_callback();
      mask Callback outputWidth: bus_expand_callback();
      mask Callback outputToWorkspace: bus_expand_callback();
  rep_addra : Subsystem  AncestorBlock=casper_library_bus/bus_replicate  mask(bus_replicate){replication=16, latency=9, misc=off, implementation=core}
      mask prompts: replication "replication factor"; misc "misc support"; implementation "delay implementation"
      mask help: Output bus formed by replicating input bus a number of times
      mask init:
      | bus_replicate_init(gcb, ...
      |     'replication', replication, ...
      |     'latency', latency, ...
      |     'implementation', implementation, ...
      |     'misc', misc);
      mask TypeOptions implementation: core | behavioral
  rep_addrb : Subsystem  AncestorBlock=casper_library_bus/bus_replicate  mask(bus_replicate){replication=16, latency=9, misc=off, implementation=core}
      mask prompts: replication "replication factor"; misc "misc support"; implementation "delay implementation"
      mask help: Output bus formed by replicating input bus a number of times
      mask init:
      | bus_replicate_init(gcb, ...
      |     'replication', replication, ...
      |     'latency', latency, ...
      |     'implementation', implementation, ...
      |     'misc', misc);
      mask TypeOptions implementation: core | behavioral
  rep_wea : Subsystem  AncestorBlock=casper_library_bus/bus_replicate  mask(bus_replicate){replication=16, latency=0, misc=off, implementation=behavioral}
      mask prompts: replication "replication factor"; misc "misc support"; implementation "delay implementation"
      mask help: Output bus formed by replicating input bus a number of times
      mask init:
      | bus_replicate_init(gcb, ...
      |     'replication', replication, ...
      |     'latency', latency, ...
      |     'implementation', implementation, ...
      |     'misc', misc);
      mask TypeOptions implementation: core | behavioral
  rep_web : Subsystem  AncestorBlock=casper_library_bus/bus_replicate  mask(bus_replicate){replication=16, latency=0, misc=off, implementation=behavioral}
      mask prompts: replication "replication factor"; misc "misc support"; implementation "delay implementation"
      mask help: Output bus formed by replicating input bus a number of times
      mask init:
      | bus_replicate_init(gcb, ...
      |     'replication', replication, ...
      |     'latency', latency, ...
      |     'implementation', implementation, ...
      |     'misc', misc);
      mask TypeOptions implementation: core | behavioral
  A : Outport
  B : Outport  Port=2
  wires:
  addra/1 -> rep_addra/1(in)
  dina/1 -> ddina/1
  wea/1 -> rep_wea/1(in)
  addrb/1 -> rep_addrb/1(in)
  dinb/1 -> ddinb/1
  web/1 -> rep_web/1(in)
  rep_addra/1(out) -> debus_addra/1(bus_in)
  ddina/1 -> debus_dina/1(bus_in)
  rep_wea/1(out) -> debus_wea/1(bus_in)
  rep_addrb/1(out) -> debus_addrb/1(bus_in)
  ddinb/1 -> debus_dinb/1(bus_in)
  rep_web/1(out) -> debus_web/1(bus_in)
  debus_addra/1(msb_out16) -> bram0/1
  debus_dina/1(msb_out16) -> bram0/2
  debus_wea/1(msb_out16) -> bram0/3
  debus_addrb/1(msb_out16) -> bram0/4
  debus_dinb/1(msb_out16) -> bram0/5
  debus_web/1(msb_out16) -> bram0/6
  debus_addra/2(out15) -> bram1/1
  debus_dina/2(out15) -> bram1/2
  debus_wea/2(out15) -> bram1/3
  debus_addrb/2(out15) -> bram1/4
  debus_dinb/2(out15) -> bram1/5
  debus_web/2(out15) -> bram1/6
  debus_addra/3(out14) -> bram2/1
  debus_dina/3(out14) -> bram2/2
  debus_wea/3(out14) -> bram2/3
  debus_addrb/3(out14) -> bram2/4
  debus_dinb/3(out14) -> bram2/5
  debus_web/3(out14) -> bram2/6
  debus_addra/4(out13) -> bram3/1
  debus_dina/4(out13) -> bram3/2
  debus_wea/4(out13) -> bram3/3
  debus_addrb/4(out13) -> bram3/4
  debus_dinb/4(out13) -> bram3/5
  debus_web/4(out13) -> bram3/6
  debus_addra/5(out12) -> bram4/1
  debus_dina/5(out12) -> bram4/2
  debus_wea/5(out12) -> bram4/3
  debus_addrb/5(out12) -> bram4/4
  debus_dinb/5(out12) -> bram4/5
  debus_web/5(out12) -> bram4/6
  debus_addra/6(out11) -> bram5/1
  debus_dina/6(out11) -> bram5/2
  debus_wea/6(out11) -> bram5/3
  debus_addrb/6(out11) -> bram5/4
  debus_dinb/6(out11) -> bram5/5
  debus_web/6(out11) -> bram5/6
  debus_addra/7(out10) -> bram6/1
  debus_dina/7(out10) -> bram6/2
  debus_wea/7(out10) -> bram6/3
  debus_addrb/7(out10) -> bram6/4
  debus_dinb/7(out10) -> bram6/5
  debus_web/7(out10) -> bram6/6
  debus_addra/8(out9) -> bram7/1
  debus_dina/8(out9) -> bram7/2
  debus_wea/8(out9) -> bram7/3
  debus_addrb/8(out9) -> bram7/4
  debus_dinb/8(out9) -> bram7/5
  debus_web/8(out9) -> bram7/6
  debus_addra/9(out8) -> bram8/1
  debus_dina/9(out8) -> bram8/2
  debus_wea/9(out8) -> bram8/3
  debus_addrb/9(out8) -> bram8/4
  debus_dinb/9(out8) -> bram8/5
  debus_web/9(out8) -> bram8/6
  debus_addra/10(out7) -> bram9/1
  debus_dina/10(out7) -> bram9/2
  debus_wea/10(out7) -> bram9/3
  debus_addrb/10(out7) -> bram9/4
  debus_dinb/10(out7) -> bram9/5
  debus_web/10(out7) -> bram9/6
  debus_addra/11(out6) -> bram10/1
  debus_dina/11(out6) -> bram10/2
  debus_wea/11(out6) -> bram10/3
  debus_addrb/11(out6) -> bram10/4
  debus_dinb/11(out6) -> bram10/5
  debus_web/11(out6) -> bram10/6
  debus_addra/12(out5) -> bram11/1
  debus_dina/12(out5) -> bram11/2
  debus_wea/12(out5) -> bram11/3
  debus_addrb/12(out5) -> bram11/4
  debus_dinb/12(out5) -> bram11/5
  debus_web/12(out5) -> bram11/6
  debus_addra/13(out4) -> bram12/1
  debus_dina/13(out4) -> bram12/2
  debus_wea/13(out4) -> bram12/3
  debus_addrb/13(out4) -> bram12/4
  debus_dinb/13(out4) -> bram12/5
  debus_web/13(out4) -> bram12/6
  debus_addra/14(out3) -> bram13/1
  debus_dina/14(out3) -> bram13/2
  debus_wea/14(out3) -> bram13/3
  debus_addrb/14(out3) -> bram13/4
  debus_dinb/14(out3) -> bram13/5
  debus_web/14(out3) -> bram13/6
  debus_addra/15(out2) -> bram14/1
  debus_dina/15(out2) -> bram14/2
  debus_wea/15(out2) -> bram14/3
  debus_addrb/15(out2) -> bram14/4
  debus_dinb/15(out2) -> bram14/5
  debus_web/15(out2) -> bram14/6
  debus_addra/16(lsb_out1) -> bram15/1
  debus_dina/16(lsb_out1) -> bram15/2
  debus_wea/16(lsb_out1) -> bram15/3
  debus_addrb/16(lsb_out1) -> bram15/4
  debus_dinb/16(lsb_out1) -> bram15/5
  debus_web/16(lsb_out1) -> bram15/6
  bram0/1 -> A_bussify/1(in1)
  bram0/2 -> B_bussify/1(in1)
  bram1/1 -> A_bussify/2(in2)
  bram1/2 -> B_bussify/2(in2)
  bram2/1 -> A_bussify/3(in3)
  bram2/2 -> B_bussify/3(in3)
  bram3/1 -> A_bussify/4(in4)
  bram3/2 -> B_bussify/4(in4)
  bram4/1 -> A_bussify/5(in5)
  bram4/2 -> B_bussify/5(in5)
  bram5/1 -> A_bussify/6(in6)
  bram5/2 -> B_bussify/6(in6)
  bram6/1 -> A_bussify/7(in7)
  bram6/2 -> B_bussify/7(in7)
  bram7/1 -> A_bussify/8(in8)
  bram7/2 -> B_bussify/8(in8)
  bram8/1 -> A_bussify/9(in9)
  bram8/2 -> B_bussify/9(in9)
  bram9/1 -> A_bussify/10(in10)
  bram9/2 -> B_bussify/10(in10)
  bram10/1 -> A_bussify/11(in11)
  bram10/2 -> B_bussify/11(in11)
  bram11/1 -> A_bussify/12(in12)
  bram11/2 -> B_bussify/12(in12)
  bram12/1 -> A_bussify/13(in13)
  bram12/2 -> B_bussify/13(in13)
  bram13/1 -> A_bussify/14(in14)
  bram13/2 -> B_bussify/14(in14)
  bram14/1 -> A_bussify/15(in15)
  bram14/2 -> B_bussify/15(in15)
  bram15/1 -> A_bussify/16(in16)
  bram15/2 -> B_bussify/16(in16)
  A_bussify/1(bus_out) -> A/1
  B_bussify/1(bus_out) -> B/1
